simulink model "zoom_adc_single_pol_no_pfb"  (Simulink 7.8)
settings: Solver=FixedStepDiscrete  SolverMode=SingleTasking  StartTime=0.0  StopTime=4096  RelTol=1e-3
libraries: xbsIndex_r4 x331, xps_library x33, casper_library_misc x29, casper_library_delays x2, xbsReplacements_r4 x2, simulink x1, gavrt_library x1, casper_library_sources x1
(part 1 of 4)
settings Model: Creator=jayanth  LastModifiedBy=dprice  ModifiedComment=dprice -- Mon Apr 22 12:22:19 2013 -- Version 1.134
settings Model: ModifiedHistory=|
  | dprice -- Mon Apr 22 12:22:19 2013 -- Version 1.134
  | 
  | 
  | dprice -- Mon Apr 22 12:19:46 2013 -- Version 1.133
  | 
  | Removing PFB to see what happens
  | 
  | dprice -- Mon Apr 22 11:47:52 2013 -- Version 1.132
  | 
  | Trying pfb_fir_real
  | 
  | dprice -- Mon Apr 22 10:52:06 2013 -- Version 1.131
  | 
  | Reworked packetization part
  | 
  | dprice -- Mon Apr 22 08:09:55 2013 -- Version 1.130
  | 
  | Changed PFB window to flat top, 8 taps
  | 
  | dprice -- Sun Apr 21 17:53:15 2013 -- Version 1.129
  | 
  | Happy with the design. Need to be careful with bit overflows however, make sure fft_shift and cplx_quant_gain are set to reasonable levels using the snap blocks.
  | 
  | dprice -- Sun Apr 21 16:38:47 2013 -- Version 1.128
  | 
  | Please god work
  | 
  | dprice -- Sun Apr 21 16:02:02 2013 -- Version 1.127
  | 
  | Arrgghhhh
  | 
  | dprice -- Sun Apr 21 15:53:36 2013 -- Version 1.126
  | 
  | Trying new convert blocks
  | 
  | dprice -- Sun Apr 21 15:21:10 2013 -- Version 1.125
  | 
  | Added in digital noise source
  | 
  | dprice -- Sun Apr 21 14:43:26 2013 -- Version 1.124
  | 
  | Just work goddamit
  | 
  | dprice -- Sun Apr 21 13:10:26 2013 -- Version 1.123
  | 
  | Added unsigned snap block to quant
  | 
  | dprice -- Sun Apr 21 12:06:49 2013 -- Version 1.122
  | 
  | 
  | dprice -- Sun Apr 21 11:54:51 2013 -- Version 1.121
  | 
  | Changed over to quant block, added snap_quant for debugging
  | 
  | dprice -- Sun Apr 21 11:40:57 2013 -- Version 1.120
  | 
  | 
  | dprice -- Sun Apr 21 10:53:59 2013 -- Version 1.119
  | 
  | pkt_en going high now resets the packet counter in the header, and resets the 10 GbE core.
  | 
  | dprice -- Sat Apr 20 15:05:59 2013 -- Version 1.118
  | 
  | Fixed slice bug
  | 
  | dprice -- Sat Apr 20 14:12:55 2013 -- Version 1.117
  | 
  | Added a snap block after the quant
  | 
  | dprice -- Sat Apr 20 14:02:46 2013 -- Version 1.116
  | 
  | Trying to stop malformed packet being sent. Added in lots of pkt_en checks, so data is only valid when pkt_en is high
  | 
  | dprice -- Fri Apr 19 10:47:57 2013 -- Version 1.115
  | 
  | Switching to black boxed fft
settings DebuggingCC: FcnCallInpInsideContextMsg=Use local settings

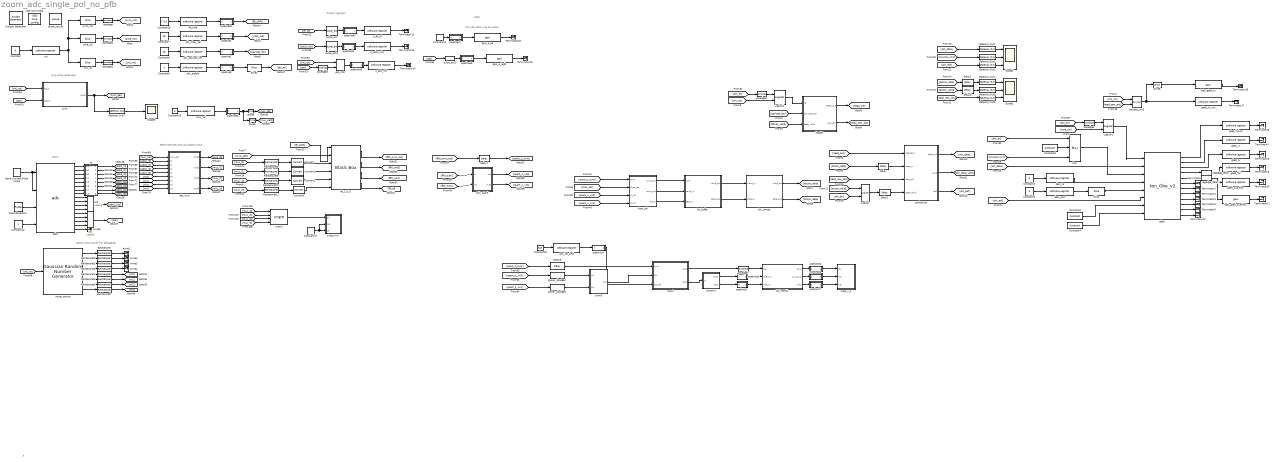
== system zoom_adc_single_pol_no_pfb ==
  note: Sync pulse reset logic
  note: ADC0
  note: Output registers
  note: this sets GPIO A as an output
  note: GPIO
  note: a
  note: Digital noise source (for debugging)
  note: Select between ADC and digital noise
  " System Generator" : xbsIndex_r4/ System Generator  Tag=genX  xilinxfamily=virtex5  part=xc5vsx95t  speed=-1  package=ff1136  synthesis_tool=XST  clock_wrapper=Clock Enables  directory=./zoom_adc_single_pol2/sysgen  proj_type=Project Navigator  Synth_file=XST Defaults  Impl_file=ISE Defaults  testbench=off  simulink_period=1  sysclk_period=5  dcm_input_clock_period=100  incr_netlist=off  trim_vbits=Everywhere in SubSystem  dbl_ovrd=According to Block Masks  core_generation=According to Block Masks  run_coregen=off  deprecated_control=off  eval_field=0  block_type=sysgen
  Arnie{1..4,9} (5 alike) : Terminator
  Band-Limited White Noise : simulink/Sources/Band-Limited White Noise  Cov=[0.1]  Ts=0.1  seed=[23341]
  Constant{,1..2,13} (4 alike) : Constant  Value=0
  Constant10 : Constant
  Constant14 : xbsIndex_r4/Constant  const=0  gui_display_data_type=Boolean  arith_type=Boolean  n_bits=16  bin_pt=14  preci_type=Single  exp_width=8  frac_width=24  explicit_period=on  period=1  equ=P=C  opselect=C  inp2=PCIN>>17  opr=+  inp1=P  carry=CIN  dbl_ovrd=off  block_type=constant
  Constant16 : Constant  Value=512
  Constant17 : xbsIndex_r4/Constant  const=1  gui_display_data_type=Boolean  arith_type=Boolean  n_bits=16  bin_pt=14  preci_type=Single  exp_width=8  frac_width=24  explicit_period=on  period=1  equ=P=C  opselect=C  inp2=PCIN>>17  opr=+  inp1=P  carry=CIN  dbl_ovrd=off  block_type=constant
  Constant18 : Constant  Value=2^5-1
  Constant3 : xbsIndex_r4/Constant  const=1  gui_display_data_type=Boolean  arith_type=Boolean  n_bits=16  bin_pt=14  preci_type=Single  exp_width=8  frac_width=24  explicit_period=on  period=1  equ=P=C  opselect=C  inp2=PCIN>>17  opr=+  inp1=P  carry=CIN  dbl_ovrd=off  block_type=constant
  Constant4 : xbsIndex_r4/Constant  const=0  gui_display_data_type=Boolean  arith_type=Boolean  n_bits=16  bin_pt=14  preci_type=Single  exp_width=8  frac_width=24  explicit_period=on  period=1  equ=P=C  opselect=C  inp2=PCIN>>17  opr=+  inp1=P  carry=CIN  dbl_ovrd=off  block_type=constant
  Constant5 : Constant
  Constant6 : xbsIndex_r4/Constant  const=0  gui_display_data_type=Boolean  arith_type=Boolean  n_bits=16  bin_pt=14  preci_type=Single  exp_width=8  frac_width=24  explicit_period=off  period=1  equ=P=C  opselect=C  inp2=PCIN>>17  opr=+  inp1=P  carry=CIN  dbl_ovrd=off  block_type=constant
  Constant7 : Constant  Value=15
  Constant8 : Constant  Value=16
  Convert{,1..3} (4 alike) : xbsIndex_r4/Convert  gui_display_data_type=Fixed-point  arith_type="Signed  (2's comp)"  n_bits=18  bin_pt=0  float_type=Single  exp_bits=8  fraction_bits=24  quantization=Truncate  overflow=Wrap  en=off  latency=0  dbl_ovrd=off  pipeline=off  block_type=convert
  Delay{,1..3} (4 alike) : xbsIndex_r4/Delay  rst=off  infoeditControl=Selection of Reset will increase slice count due to use of real FFs and instead of SRLs  en=off  latency=1  dbl_ovrd=off  reg_retiming=off  block_type=delay
  Delay6 : xbsIndex_r4/Delay  rst=off  infoeditControl=Selection of Reset will increase slice count due to use of real FFs and instead of SRLs  en=off  latency=4  dbl_ovrd=off  reg_retiming=off  block_type=delay
  Delay7 : xbsIndex_r4/Delay  rst=off  infoeditControl=Selection of Reset will increase slice count due to use of real FFs and instead of SRLs  en=off  latency=6  dbl_ovrd=off  reg_retiming=off  block_type=delay
  Gateway Out{1..7} (7 alike) : xbsIndex_r4/Gateway Out  inherit_from_input=off  hdl_port=on  timing_constraint=None  locs_specified=off  LOCs={}  UseAsDAC=off  DACChannel='1'  block_type=gatewayout
      settings: infoedit="Gateway out block.  Converts Xilinx fixed-point or floating-point type inputs into ouputs of type Simulink integer, single, double, or fixed point.<br><br>Hardware notes:  In hardware these blocks become top level output ports or are discarded, depending on how they are configured."
  Logical : xbsIndex_r4/Logical  logical_function=AND  inputs=2  en=off  latency=0  precision=Full  arith_type=Unsigned  n_bits=16  bin_pt=0  align_bp=on  dbl_ovrd=off  block_type=logical
  Logical1 : xbsIndex_r4/Logical  logical_function=OR  inputs=2  en=off  latency=0  precision=Full  arith_type=Unsigned  n_bits=16  bin_pt=0  align_bp=on  dbl_ovrd=off  block_type=logical
  Logical2 : xbsIndex_r4/Logical  logical_function=OR  inputs=2  en=off  latency=0  precision=Full  arith_type=Unsigned  n_bits=16  bin_pt=0  align_bp=on  dbl_ovrd=off  block_type=logical
  Mux : xbsIndex_r4/Mux  inputs=2  en=off  latency=0  precision=Full  arith_type=Unsigned  n_bits=16  bin_pt=14  quantization=Truncate  overflow=Wrap  dbl_ovrd=off  block_type=mux
  Pulse Generator : DiscretePulseGenerator  PulseType=Time based  Period=10  PulseWidth=5
  Reinterpret{,1..7} (8 alike) : xbsIndex_r4/Reinterpret  force_arith_type=on  arith_type="Signed  (2's comp)"  force_bin_pt=on  bin_pt=7  block_type=reinterpret
  Reinterpret{8..11} (4 alike) : xbsIndex_r4/Reinterpret  force_arith_type=on  arith_type="Signed  (2's comp)"  force_bin_pt=on  bin_pt=0  block_type=reinterpret
  Scope1 : Scope  NumInputPorts=3
      settings: TimeRange=290.1459854014598  YMin=9.44473e+21~0~-1  YMax=3.5e+38~1~1
  Scope2 : Scope  NumInputPorts=3
      settings: TimeRange=290.1459854014598  YMin=0~0~0  YMax=1.2e+18~1~1
  Scope3 : Scope
      settings: SaveName=ScopeData6
  Slice : xbsIndex_r4/Slice  nbits=16  boolean_output=off  mode=Lower Bit Location + Width  bit1=0  base1=MSB of Input  bit0=0  base0=LSB of Input  dbl_ovrd=off  block_type=slice
  Slice1 : xbsIndex_r4/Slice  nbits=1  boolean_output=on  mode=Lower Bit Location + Width  bit1=1  base1=MSB of Input  bit0=0  base0=LSB of Input  dbl_ovrd=off  block_type=slice
      settings: block_version=10.1.3
  Slice3 : xbsIndex_r4/Slice  nbits=1  boolean_output=on  mode=Lower Bit Location + Width  bit1=0  base1=MSB of Input  bit0=0  base0=LSB of Input  dbl_ovrd=off  block_type=slice
      settings: infoedit="Extracts a given range of bits from each input sample and presents it at the output.  The output type is ordinarily unsigned with binary point at zero, but can be Boolean when the slice is one bit wide.<P><P>Hardware notes: In hardware this block costs nothing."  block_version=10.1.3
  Slice5 : xbsIndex_r4/Slice  nbits=1  boolean_output=on  mode=Lower Bit Location + Width  bit1=0  base1=MSB of Input  bit0=1  base0=LSB of Input  dbl_ovrd=off  block_type=slice
      settings: block_version=10.1.3
  Slice6 : xbsIndex_r4/Slice  nbits=1  boolean_output=on  mode=Lower Bit Location + Width  bit1=0  base1=MSB of Input  bit0=15  base0=LSB of Input  dbl_ovrd=off  block_type=slice
      settings: block_version=10.1.3
  Terminator{,1..19} (20 alike) : Terminator
  XSG core config : xps_library/XSG core config  Tag=xps:xsg  hw_sys=ROACH:sx95t  ibob_linux=off  clk_src=adc0_clk  iBOB_clk_src=sys_clk  BEE2_ctrl_clk_src=sys_clk  BEE2_usr_clk_src=sys_clk  ROACH_clk_src=adc0_clk  ROACH2_clk_src=adc0_clk  CORR_clk_src=sys_clk  gpio_clk_io_group=iBOB:sma  gpio_clk_bit_index=0  clk_rate=200  sample_period=1  synthesis_tool=XST  mpc_type=powerpc440_ext
  acc_len_test : xps_library/software register  Tag=xps:sw_reg  io_dir=From Processor  arith_type=Unsigned  bitwidth=32  bin_pt=0  sample_period=1  gw_name=zoom_adc_single_pol2_acc_len_test_user_data_out
      link override coarse_r01_acc_len_user_data_out: arith_type=Unsigned  n_bits=32  bin_pt=0  period=1
      link override coarse_r02_acc_len_user_data_out: arith_type=Unsigned  n_bits=32  bin_pt=0  period=1
      link override coarse_r03_acc_len_user_data_out: arith_type=Unsigned  n_bits=32  bin_pt=0  period=1
      link override coarse_r04_acc_len_user_data_out: arith_type=Unsigned  n_bits=32  bin_pt=0  period=1
      link override coarse_r06_acc_len_user_data_out: arith_type=Unsigned  n_bits=32  bin_pt=0  period=1
      link override coarse_r07_acc_len_user_data_out: arith_type=Unsigned  n_bits=32  bin_pt=0  period=1
      link override coarse_r08_acc_len_user_data_out: arith_type=Unsigned  n_bits=32  bin_pt=0  period=1
      link override coarse_r09_acc_len_user_data_out: arith_type=Unsigned  n_bits=32  bin_pt=0  period=1
      link override coarse_r10_acc_len_user_data_out: arith_type=Unsigned  n_bits=32  bin_pt=0  period=1
      link override coarse_r12_acc_len_user_data_out: arith_type=Unsigned  n_bits=32  bin_pt=0  period=1
      link override convert: arith_type=Unsigned  n_bits=32  bin_pt=0
      link override qdr_single_r01_acc_len_coarse_user_data_out: arith_type=Unsigned  n_bits=32  bin_pt=0  period=1
      link override qdr_single_r02_acc_len_coarse_user_data_out: arith_type=Unsigned  n_bits=32  bin_pt=0  period=1
      link override qdr_single_r04_acc_len_coarse_user_data_out: arith_type=Unsigned  n_bits=32  bin_pt=0  period=1
      link override r_spec_2048_r102_acc_len_user_data_out: arith_type=Unsigned  n_bits=32  bin_pt=0  period=1
      link override r_spec_2048_r102_nosim_acc_len_user_data_out: arith_type=Unsigned  n_bits=32  bin_pt=0  period=1
      link override r_spec_2048_r103_acc_len_user_data_out: arith_type=Unsigned  n_bits=32  bin_pt=0  period=1
      link override r_spec_2048_r104_acc_len_user_data_out: arith_type=Unsigned  n_bits=32  bin_pt=0  period=1
      link override r_spec_2048_r104_test0_acc_len_user_data_out: arith_type=Unsigned  n_bits=32  bin_pt=0  period=1
      link override r_spec_2048_r104_test_acc_len_user_data_out: arith_type=Unsigned  n_bits=32  bin_pt=0  period=1
      link override r_spec_2048_r105_acc_len_user_data_out: arith_type=Unsigned  n_bits=32  bin_pt=0  period=1
      link override r_spec_test0_acc_len_user_data_out: arith_type=Unsigned  n_bits=32  bin_pt=0  period=1
      link override r_spec_test4_acc_len_user_data_out: arith_type=Unsigned  n_bits=32  bin_pt=0  period=1
      link override roach_spec_acc_len_user_data_out: arith_type=Unsigned  n_bits=32  bin_pt=0  period=1
      link override roach_spec_no10gbe_acc_len_user_data_out: arith_type=Unsigned  n_bits=32  bin_pt=0  period=1
      link override roach_spec_rst_sync_cnt_user_data_out: arith_type=Unsigned  n_bits=32  bin_pt=0  period=1
      link override toast_256ch_16bit_r02_acc_len_user_data_out: arith_type=Unsigned  n_bits=32  bin_pt=0  period=1
      link override toast_256ch_16bit_r04_acc_len_user_data_out: arith_type=Unsigned  n_bits=32  bin_pt=0  period=1
      link override toast_256ch_16bit_r05_acc_len_user_data_out: arith_type=Unsigned  n_bits=32  bin_pt=0  period=1
  adc0 : xps_library/adc  Tag=xps:adc  adc_brd=adc0  adc_clk_rate=800  adc_interleave=off  sample_period=1
  adc_mux : Subsystem
  bit_buffer : Subsystem
  bit_concat : Subsystem
  buf_reord1 : Subsystem  mask{buf_len_bits=3}
      mask prompts: buf_len_bits "Buffer length (2^N)"
      mask help: Concatenate signals together for snap block
  chan_sel : Subsystem
  cnt_rst : xbsIndex_r4/Slice  nbits=1  boolean_output=on  mode=Lower Bit Location + Width  bit1=0  base1=MSB of Input  bit0=0  base0=LSB of Input  dbl_ovrd=off  block_type=slice
  core_rst : xbsIndex_r4/Slice  nbits=1  boolean_output=on  mode=Lower Bit Location + Width  bit1=0  base1=MSB of Input  bit0=1  base0=LSB of Input  dbl_ovrd=off  block_type=slice
  cplx_quant : Subsystem  AncestorBlock=hipsr_library/quant
  cram1 : gavrt_library/cram  num_slice=4
  cram5 : Subsystem  AncestorBlock=gavrt_library/cram  mask(cram){num_slice=2}
      mask prompts: num_slice "Number of slices"
      mask help: Concatenates inputs together after forcing binary point to zero.
      mask init:
      | cram_init(gcb, ...
      |     'num_slice', num_slice);
  dest_ip : xps_library/software register  Tag=xps:sw_reg  io_dir=From Processor  arith_type=Unsigned  bitwidth=32  bin_pt=0  sample_period=1  gw_name=zoom_adc_single_pol2_dest_ip_user_data_out
      link override hipsr_tgbe3_dest_ip_user_data_out: arith_type=Unsigned  n_bits=32  bin_pt=0  period=1
  dest_port : xps_library/software register  Tag=xps:sw_reg  io_dir=From Processor  arith_type=Unsigned  bitwidth=32  bin_pt=0  sample_period=1  gw_name=zoom_adc_single_pol2_dest_port_user_data_out
      link override hipsr_tgbe3_dest_port_user_data_out: arith_type=Unsigned  n_bits=32  bin_pt=0  period=1
  fft_11x_0 : xbsIndex_r4/Black Box  init_code=fft_32pt_11x_core_config  sim_method=Inactive  verbose=off  block_type=blackbox2
  fft_shift : xps_library/software register  Tag=xps:sw_reg  io_dir=From Processor  arith_type=Unsigned  bitwidth=32  bin_pt=0  sample_period=1  gw_name=zoom_adc_single_pol2_fft_shift_user_data_out
      link override coarse_r01_acc_len_user_data_out: arith_type=Unsigned  n_bits=32  bin_pt=0  period=1
      link override coarse_r02_acc_len_user_data_out: arith_type=Unsigned  n_bits=32  bin_pt=0  period=1
      link override coarse_r03_acc_len_user_data_out: arith_type=Unsigned  n_bits=32  bin_pt=0  period=1
      link override coarse_r04_acc_len_user_data_out: arith_type=Unsigned  n_bits=32  bin_pt=0  period=1
      link override coarse_r06_acc_len_user_data_out: arith_type=Unsigned  n_bits=32  bin_pt=0  period=1
      link override coarse_r07_acc_len_user_data_out: arith_type=Unsigned  n_bits=32  bin_pt=0  period=1
      link override coarse_r08_acc_len_user_data_out: arith_type=Unsigned  n_bits=32  bin_pt=0  period=1
      link override coarse_r09_acc_len_user_data_out: arith_type=Unsigned  n_bits=32  bin_pt=0  period=1
      link override coarse_r10_acc_len_user_data_out: arith_type=Unsigned  n_bits=32  bin_pt=0  period=1
      link override coarse_r12_acc_len_user_data_out: arith_type=Unsigned  n_bits=32  bin_pt=0  period=1
      link override convert: arith_type=Unsigned  n_bits=32  bin_pt=0
      link override qdr_single_r01_acc_len_coarse_user_data_out: arith_type=Unsigned  n_bits=32  bin_pt=0  period=1
      link override qdr_single_r02_acc_len_coarse_user_data_out: arith_type=Unsigned  n_bits=32  bin_pt=0  period=1
      link override qdr_single_r04_acc_len_coarse_user_data_out: arith_type=Unsigned  n_bits=32  bin_pt=0  period=1
      link override r_spec_2048_r102_acc_len_user_data_out: arith_type=Unsigned  n_bits=32  bin_pt=0  period=1
      link override r_spec_2048_r102_nosim_acc_len_user_data_out: arith_type=Unsigned  n_bits=32  bin_pt=0  period=1
      link override r_spec_2048_r103_acc_len_user_data_out: arith_type=Unsigned  n_bits=32  bin_pt=0  period=1
      link override r_spec_2048_r104_acc_len_user_data_out: arith_type=Unsigned  n_bits=32  bin_pt=0  period=1
      link override r_spec_2048_r104_test0_acc_len_user_data_out: arith_type=Unsigned  n_bits=32  bin_pt=0  period=1
      link override r_spec_2048_r104_test_acc_len_user_data_out: arith_type=Unsigned  n_bits=32  bin_pt=0  period=1
      link override r_spec_2048_r105_acc_len_user_data_out: arith_type=Unsigned  n_bits=32  bin_pt=0  period=1
      link override r_spec_test0_acc_len_user_data_out: arith_type=Unsigned  n_bits=32  bin_pt=0  period=1
      link override r_spec_test4_acc_len_user_data_out: arith_type=Unsigned  n_bits=32  bin_pt=0  period=1
      link override roach_spec_acc_len_user_data_out: arith_type=Unsigned  n_bits=32  bin_pt=0  period=1
      link override roach_spec_no10gbe_acc_len_user_data_out: arith_type=Unsigned  n_bits=32  bin_pt=0  period=1
      link override roach_spec_rst_sync_cnt_user_data_out: arith_type=Unsigned  n_bits=32  bin_pt=0  period=1
      link override toast_256ch_16bit_r02_acc_len_user_data_out: arith_type=Unsigned  n_bits=32  bin_pt=0  period=1
      link override toast_256ch_16bit_r04_acc_len_user_data_out: arith_type=Unsigned  n_bits=32  bin_pt=0  period=1
      link override toast_256ch_16bit_r05_acc_len_user_data_out: arith_type=Unsigned  n_bits=32  bin_pt=0  period=1
  gbe0 : xps_library/ten_Gbe_v2  Tag=xps:tengbe_v2  port=0  rx_dist_ram=off  large_frames=on  show_param=off  pre_emph=3  swing=800  fab_en=on  fab_mac=hex2dec('123456780000')  fab_ip=192*(2^24) + 168*(2^16) + 5*(2^8) + 20*(2^0)  fab_udp=10000  fab_gate=1  cpu_rx_en=on  cpu_tx_en=on
  gbe0_full : xps_library/software register  Tag=xps:sw_reg  io_dir=To Processor  arith_type=Unsigned  bitwidth=32  bin_pt=0  sample_period=1  gw_name=zoom_adc_single_pol2_gbe0_full_user_data_in
      link override convert: arith_type=Unsigned  n_bits=32  bin_pt=0
  gbe0_linkup : xps_library/software register  Tag=xps:sw_reg  io_dir=To Processor  arith_type=Unsigned  bitwidth=32  bin_pt=0  sample_period=1  gw_name=zoom_adc_single_pol2_gbe0_linkup_user_data_in
      link override convert: arith_type=Unsigned  n_bits=32  bin_pt=0
  gbe0_overflow : xps_library/software register  Tag=xps:sw_reg  io_dir=To Processor  arith_type=Unsigned  bitwidth=32  bin_pt=0  sample_period=1  gw_name=zoom_adc_single_pol2_gbe0_overflow_user_data_in
      link override convert: arith_type=Unsigned  n_bits=32  bin_pt=0
  gbe0_rx : xps_library/software register  Tag=xps:sw_reg  io_dir=To Processor  arith_type=Unsigned  bitwidth=32  bin_pt=0  sample_period=1  gw_name=zoom_adc_single_pol2_gbe0_rx_user_data_in
      link override convert: arith_type=Unsigned  n_bits=32  bin_pt=0
  gbe0_tx : xps_library/software register  Tag=xps:sw_reg  io_dir=To Processor  arith_type=Unsigned  bitwidth=32  bin_pt=0  sample_period=1  gw_name=zoom_adc_single_pol2_gbe0_tx_user_data_in
      link override convert: arith_type=Unsigned  n_bits=32  bin_pt=0
  gbe0_tx_cnt : xps_library/software register  Tag=xps:sw_reg  io_dir=To Processor  arith_type=Unsigned  bitwidth=32  bin_pt=0  sample_period=1  gw_name=zoom_adc_single_pol2_gbe0_tx_cnt_user_data_in
      link override convert: arith_type=Unsigned  n_bits=32  bin_pt=0
  gpio_a_led0 : xps_library/gpio  Tag=xps:gpio  io_group=ROACH:gpioa  io_dir=out  arith_type=Boolean  bitwidth=1  bin_pt=0  bit_index=4  sample_period=1  use_single_ended=off  use_ddr=off  reg_iob=on  reg_clk_phase=0  termination=None
  gpio_a_oe : xps_library/gpio  Tag=xps:gpio  io_group=ROACH:gpioa_oe_n  io_dir=out  arith_type=Boolean  bitwidth=1  bin_pt=0  bit_index=0  sample_period=1  use_single_ended=off  use_ddr=off  reg_iob=on  reg_clk_phase=0  termination=None
  header : Subsystem
  led0_gbe0_overflow : xps_library/gpio  Tag=xps:gpio  io_group=ROACH:led  io_dir=out  arith_type=Boolean  bitwidth=1  bin_pt=0  bit_index=1  sample_period=1  use_single_ended=off  use_ddr=off  reg_iob=on  reg_clk_phase=0  termination=None
      link override convert: arith_type=Boolean  n_bits=1  bin_pt=0
  led0_gbe0_tx : xps_library/gpio  Tag=xps:gpio  io_group=ROACH:led  io_dir=out  arith_type=Boolean  bitwidth=1  bin_pt=0  bit_index=0  sample_period=1  use_single_ended=off  use_ddr=off  reg_iob=off  reg_clk_phase=0  termination=None
      link override convert: arith_type=Boolean  n_bits=1  bin_pt=0
  mux_sel : xps_library/software register  Tag=xps:sw_reg  io_dir=From Processor  arith_type=Unsigned  bitwidth=32  bin_pt=0  sample_period=1  gw_name=zoom_adc_single_pol2_mux_sel_user_data_out
      link override coarse_r01_mux_sel_user_data_out: arith_type=Unsigned  n_bits=32  bin_pt=0  period=1
      link override coarse_r02_mux_sel_user_data_out: arith_type=Unsigned  n_bits=32  bin_pt=0  period=1
      link override coarse_r03_mux_sel_user_data_out: arith_type=Unsigned  n_bits=32  bin_pt=0  period=1
      link override coarse_r04_mux_sel_user_data_out: arith_type=Unsigned  n_bits=32  bin_pt=0  period=1
      link override coarse_r06_mux_sel_user_data_out: arith_type=Unsigned  n_bits=32  bin_pt=0  period=1
      link override coarse_r07_mux_sel_user_data_out: arith_type=Unsigned  n_bits=32  bin_pt=0  period=1
      link override coarse_r08_mux_sel_user_data_out: arith_type=Unsigned  n_bits=32  bin_pt=0  period=1
      link override coarse_r09_mux_sel_user_data_out: arith_type=Unsigned  n_bits=32  bin_pt=0  period=1
      link override coarse_r10_mux_sel_user_data_out: arith_type=Unsigned  n_bits=32  bin_pt=0  period=1
      link override coarse_r12_mux_sel_user_data_out: arith_type=Unsigned  n_bits=32  bin_pt=0  period=1
      link override convert: arith_type=Unsigned  n_bits=32  bin_pt=0
      link override qdr_single_r01_mux_sel_user_data_out: arith_type=Unsigned  n_bits=32  bin_pt=0  period=1
      link override qdr_single_r02_mux_sel_user_data_out: arith_type=Unsigned  n_bits=32  bin_pt=0  period=1
      link override qdr_single_r04_mux_sel_user_data_out: arith_type=Unsigned  n_bits=32  bin_pt=0  period=1
      link override r_spec_2048_r102_cnt_rst_user_data_out: arith_type=Unsigned  n_bits=32  bin_pt=0  period=1
      link override r_spec_2048_r102_nosim_cnt_rst_user_data_out: arith_type=Unsigned  n_bits=32  bin_pt=0  period=1
      link override r_spec_2048_r103_cnt_rst_user_data_out: arith_type=Unsigned  n_bits=32  bin_pt=0  period=1
      link override r_spec_2048_r104_cnt_rst_user_data_out: arith_type=Unsigned  n_bits=32  bin_pt=0  period=1
      link override r_spec_2048_r104_test0_cnt_rst_user_data_out: arith_type=Unsigned  n_bits=32  bin_pt=0  period=1
      link override r_spec_2048_r104_test_cnt_rst_user_data_out: arith_type=Unsigned  n_bits=32  bin_pt=0  period=1
      link override r_spec_2048_r105_cnt_rst_user_data_out: arith_type=Unsigned  n_bits=32  bin_pt=0  period=1
      link override r_spec_test0_cnt_rst_user_data_out: arith_type=Unsigned  n_bits=32  bin_pt=0  period=1
      link override r_spec_test4_cnt_rst_user_data_out: arith_type=Unsigned  n_bits=32  bin_pt=0  period=1
      link override roach_spec_no10gbe_sync_cnt_rst_user_data_out: arith_type=Unsigned  n_bits=32  bin_pt=0  period=1
      link override roach_spec_rst_cnt_user_data_out: arith_type=Unsigned  n_bits=32  bin_pt=0  period=1
      link override roach_spec_rst_sync_cnt_user_data_out: arith_type=Unsigned  n_bits=32  bin_pt=0  period=1
      link override toast_256ch_16bit_r02_cnt_rst_user_data_out: arith_type=Unsigned  n_bits=32  bin_pt=0  period=1
      link override toast_256ch_16bit_r04_cnt_rst_user_data_out: arith_type=Unsigned  n_bits=32  bin_pt=0  period=1
      link override toast_256ch_16bit_r05_cnt_rst_user_data_out: arith_type=Unsigned  n_bits=32  bin_pt=0  period=1
  negedge_delay : casper_library_misc/negedge_delay  pulse_len=2^20
  negedge_delay1 : casper_library_misc/negedge_delay  pulse_len=2^20
  noise_source : casper_library_sources/Gaussian Random Number Generator
  o_adc0_clip : xps_library/software register  Tag=xps:sw_reg  io_dir=To Processor  arith_type=Unsigned  bitwidth=32  bin_pt=0  sample_period=1  gw_name=zoom_adc_single_pol2_o_adc0_clip_user_data_in
      link override convert: arith_type=Unsigned  n_bits=32  bin_pt=0
  o_fft_of : xps_library/software register  Tag=xps:sw_reg  io_dir=To Processor  arith_type=Unsigned  bitwidth=32  bin_pt=0  sample_period=1  gw_name=zoom_adc_single_pol2_o_fft_of_user_data_in
      link override convert: arith_type=Unsigned  n_bits=32  bin_pt=0
  o_pps_cnt : xps_library/software register  Tag=xps:sw_reg  io_dir=To Processor  arith_type=Unsigned  bitwidth=32  bin_pt=0  sample_period=1  gw_name=zoom_adc_single_pol2_o_pps_cnt_user_data_in
      link override convert: arith_type=Unsigned  n_bits=32  bin_pt=0
  or0 : xbsIndex_r4/Logical  logical_function=OR  inputs=4  en=off  latency=2  precision=Full  arith_type=Unsigned  n_bits=16  bin_pt=0  align_bp=off  dbl_ovrd=off  block_type=logical
  or1 : xbsIndex_r4/Logical  logical_function=OR  inputs=4  en=off  latency=2  precision=Full  arith_type=Unsigned  n_bits=16  bin_pt=0  align_bp=off  dbl_ovrd=off  block_type=logical
  packetizer : Subsystem
  payload_cnt5 : xbsIndex_r4/Counter  cnt_type=Free Running  cnt_to=0  operation=Up  start_count=0  cnt_by_val=1  arith_type=Unsigned  n_bits=32  bin_pt=0  load_pin=off  rst=on  en=on  explicit_period=on  period=1  dbl_ovrd=off  use_behavioral_HDL=off  implementation=Fabric  use_rpm=on  block_type=counter
      settings: xl_area=[0, 0, 0, 0, 0, 0, 0]
  pcore_nar_fft : xps_library/pcore  Tag=xps:pcore  pcore_path=./bb/fft_32pt_11x_core/fft_32pt_11x_core.ngc
  pipeline1 : Subsystem  AncestorBlock=casper_library_delays/pipeline  mask(pipeline){latency=1}
      mask help:
      | An explicitly laid-out delay line to avoid mapping to SRL16s
      | for use in pipelining to help achieve timing closure.
      mask init:
      | pipeline_init(gcb,'latency',latency);
      settings: AttributesFormatString=Latency = %<latency>
      mask Display:
      | port_label('input', 1, 'd');
      | port_label('output', 1, 'q');
      | disp(['Z^-', num2str(latency)]);
  pipeline14 : Subsystem  AncestorBlock=casper_library_delays/pipeline  mask(pipeline){latency=1}
      mask help:
      | An explicitly laid-out delay line to avoid mapping to SRL16s
      | for use in pipelining to help achieve timing closure.
      mask init:
      | pipeline_init(gcb,'latency',latency);
      settings: AttributesFormatString=Latency = %<latency>
      mask Display:
      | port_label('input', 1, 'd');
      | port_label('output', 1, 'q');
      | disp(['Z^-', num2str(latency)]);
  pipeline15 : Subsystem  AncestorBlock=casper_library_delays/pipeline  mask(pipeline){latency=1}
      mask help:
      | An explicitly laid-out delay line to avoid mapping to SRL16s
      | for use in pipelining to help achieve timing closure.
      mask init:
      | pipeline_init(gcb,'latency',latency);
      settings: AttributesFormatString=Latency = %<latency>
      mask Display:
      | port_label('input', 1, 'd');
      | port_label('output', 1, 'q');
      | disp(['Z^-', num2str(latency)]);
  pipeline17 : Subsystem  AncestorBlock=casper_library_delays/pipeline  mask(pipeline){latency=1}
      mask help:
      | An explicitly laid-out delay line to avoid mapping to SRL16s
      | for use in pipelining to help achieve timing closure.
      mask init:
      | pipeline_init(gcb,'latency',latency);
      settings: AttributesFormatString=Latency = %<latency>
      mask Display:
      | port_label('input', 1, 'd');
      | port_label('output', 1, 'q');
      | disp(['Z^-', num2str(latency)]);
  pipeline18 : Subsystem  AncestorBlock=casper_library_delays/pipeline  mask(pipeline){latency=1}
      mask help:
      | An explicitly laid-out delay line to avoid mapping to SRL16s
      | for use in pipelining to help achieve timing closure.
      mask init:
      | pipeline_init(gcb,'latency',latency);
      settings: AttributesFormatString=Latency = %<latency>
      mask Display:
      | port_label('input', 1, 'd');
      | port_label('output', 1, 'q');
      | disp(['Z^-', num2str(latency)]);
  pipeline2 : Subsystem  AncestorBlock=casper_library_delays/pipeline  mask(pipeline){latency=1}
      mask help:
      | An explicitly laid-out delay line to avoid mapping to SRL16s
      | for use in pipelining to help achieve timing closure.
      mask init:
      | pipeline_init(gcb,'latency',latency);
      settings: AttributesFormatString=Latency = %<latency>
      mask Display:
      | port_label('input', 1, 'd');
      | port_label('output', 1, 'q');
      | disp(['Z^-', num2str(latency)]);
  pipeline21 : Subsystem  AncestorBlock=casper_library_delays/pipeline  mask(pipeline){latency=1}
      mask help:
      | An explicitly laid-out delay line to avoid mapping to SRL16s
      | for use in pipelining to help achieve timing closure.
      mask init:
      | pipeline_init(gcb,'latency',latency);
      settings: AttributesFormatString=Latency = %<latency>
      mask Display:
      | port_label('input', 1, 'd');
      | port_label('output', 1, 'q');
      | disp(['Z^-', num2str(latency)]);
  pipeline22 : Subsystem  AncestorBlock=casper_library_delays/pipeline  mask(pipeline){latency=1}
      mask help:
      | An explicitly laid-out delay line to avoid mapping to SRL16s
      | for use in pipelining to help achieve timing closure.
      mask init:
      | pipeline_init(gcb,'latency',latency);
      settings: AttributesFormatString=Latency = %<latency>
      mask Display:
      | port_label('input', 1, 'd');
      | port_label('output', 1, 'q');
      | disp(['Z^-', num2str(latency)]);
  pipeline28 : Subsystem  AncestorBlock=casper_library_delays/pipeline  mask(pipeline){latency=1}
      mask help:
      | An explicitly laid-out delay line to avoid mapping to SRL16s
      | for use in pipelining to help achieve timing closure.
      mask init:
      | pipeline_init(gcb,'latency',latency);
      settings: AttributesFormatString=Latency = %<latency>
      mask Display:
      | port_label('input', 1, 'd');
      | port_label('output', 1, 'q');
      | disp(['Z^-', num2str(latency)]);
  pipeline3 : Subsystem  AncestorBlock=casper_library_delays/pipeline  mask(pipeline){latency=1}
      mask help:
      | An explicitly laid-out delay line to avoid mapping to SRL16s
      | for use in pipelining to help achieve timing closure.
      mask init:
      | pipeline_init(gcb,'latency',latency);
      settings: AttributesFormatString=Latency = %<latency>
      mask Display:
      | port_label('input', 1, 'd');
      | port_label('output', 1, 'q');
      | disp(['Z^-', num2str(latency)]);
  pipeline43 : Subsystem  AncestorBlock=casper_library_delays/pipeline  mask(pipeline){latency=1}
      mask help:
      | An explicitly laid-out delay line to avoid mapping to SRL16s
      | for use in pipelining to help achieve timing closure.
      mask init:
      | pipeline_init(gcb,'latency',latency);
      settings: AttributesFormatString=Latency = %<latency>
      mask Display:
      | port_label('input', 1, 'd');
      | port_label('output', 1, 'q');
      | disp(['Z^-', num2str(latency)]);
  pipeline44 : Subsystem  AncestorBlock=casper_library_delays/pipeline  mask(pipeline){latency=1}
      mask help:
      | An explicitly laid-out delay line to avoid mapping to SRL16s
      | for use in pipelining to help achieve timing closure.
      mask init:
      | pipeline_init(gcb,'latency',latency);
      settings: AttributesFormatString=Latency = %<latency>
      mask Display:
      | port_label('input', 1, 'd');
      | port_label('output', 1, 'q');
      | disp(['Z^-', num2str(latency)]);
  pipeline46 : Subsystem  AncestorBlock=casper_library_delays/pipeline  mask(pipeline){latency=1}
      mask help:
      | An explicitly laid-out delay line to avoid mapping to SRL16s
      | for use in pipelining to help achieve timing closure.
      mask init:
      | pipeline_init(gcb,'latency',latency);
      settings: AttributesFormatString=Latency = %<latency>
      mask Display:
      | port_label('input', 1, 'd');
      | port_label('output', 1, 'q');
      | disp(['Z^-', num2str(latency)]);
  pipeline47 : Subsystem  AncestorBlock=casper_library_delays/pipeline  mask(pipeline){latency=1}
      mask help:
      | An explicitly laid-out delay line to avoid mapping to SRL16s
      | for use in pipelining to help achieve timing closure.
      mask init:
      | pipeline_init(gcb,'latency',latency);
      settings: AttributesFormatString=Latency = %<latency>
      mask Display:
      | port_label('input', 1, 'd');
      | port_label('output', 1, 'q');
      | disp(['Z^-', num2str(latency)]);
  pipeline48 : Subsystem  AncestorBlock=casper_library_delays/pipeline  mask(pipeline){latency=1}
      mask help:
      | An explicitly laid-out delay line to avoid mapping to SRL16s
      | for use in pipelining to help achieve timing closure.
      mask init:
      | pipeline_init(gcb,'latency',latency);
      settings: AttributesFormatString=Latency = %<latency>
      mask Display:
      | port_label('input', 1, 'd');
      | port_label('output', 1, 'q');
      | disp(['Z^-', num2str(latency)]);
  pipeline50 : Subsystem  AncestorBlock=casper_library_delays/pipeline  mask(pipeline){latency=1}
      mask help:
      | An explicitly laid-out delay line to avoid mapping to SRL16s
      | for use in pipelining to help achieve timing closure.
      mask init:
      | pipeline_init(gcb,'latency',latency);
      settings: AttributesFormatString=Latency = %<latency>
      mask Display:
      | port_label('input', 1, 'd');
      | port_label('output', 1, 'q');
      | disp(['Z^-', num2str(latency)]);
  pkt_chan_sel : xps_library/software register  Tag=xps:sw_reg  io_dir=From Processor  arith_type=Unsigned  bitwidth=32  bin_pt=0  sample_period=1  gw_name=zoom_adc_single_pol2_pkt_chan_sel_user_data_out
      link override hipsr_tgbe3_pkt_chan_sel_user_data_out: arith_type=Unsigned  n_bits=32  bin_pt=0  period=1
  pkt_enable : xps_library/software register  Tag=xps:sw_reg  io_dir=From Processor  arith_type=Unsigned  bitwidth=32  bin_pt=0  sample_period=1  gw_name=zoom_adc_single_pol2_pkt_enable_user_data_out
      link override hipsr_tgbe3_pkt_enable_user_data_out: arith_type=Unsigned  n_bits=32  bin_pt=0  period=1
  pkt_payload_len : xps_library/software register  Tag=xps:sw_reg  io_dir=From Processor  arith_type=Unsigned  bitwidth=32  bin_pt=0  sample_period=1  gw_name=zoom_adc_single_pol2_pkt_payload_len_user_data_out
      link override hipsr_tgbe3_pkt_payload_len_user_data_out: arith_type=Unsigned  n_bits=32  bin_pt=0  period=1
  pl : Subsystem
  posedge{,1..5,7} (7 alike) : casper_library_misc/posedge  Tag=Rising Edge Detector
  power_dsp48e2 : casper_library_misc/power_dsp48e  bitwidth=8
      link override slice_imag: nbits=bitwidth  boolean_output=off  mode=Lower Bit Location + Width  bit1=0  base1=MSB of Input  bit0=0  base0=LSB of Input  dbl_ovrd=off
      link override slice_real: nbits=bitwidth  boolean_output=off  mode=Upper Bit Location + Width  bit1=0  base1=MSB of Input  bit0=0  base0=LSB of Input  dbl_ovrd=off
      link override slice_imag: infoedit="Extracts a given range of bits from each input sample and presents it at the output.  The output type is ordinarily unsigned with binary point at zero, but can be Boolean when the slice is one bit wide.<br><br>Hardware notes: In hardware this block costs nothing."
      link override slice_real: infoedit="Extracts a given range of bits from each input sample and presents it at the output.  The output type is ordinarily unsigned with binary point at zero, but can be Boolean when the slice is one bit wide.<br><br>Hardware notes: In hardware this block costs nothing."
  power_dsp48e3 : casper_library_misc/power_dsp48e  bitwidth=8
      link override slice_imag: nbits=bitwidth  boolean_output=off  mode=Lower Bit Location + Width  bit1=0  base1=MSB of Input  bit0=0  base0=LSB of Input  dbl_ovrd=off
      link override slice_real: nbits=bitwidth  boolean_output=off  mode=Upper Bit Location + Width  bit1=0  base1=MSB of Input  bit0=0  base0=LSB of Input  dbl_ovrd=off
      link override slice_imag: infoedit="Extracts a given range of bits from each input sample and presents it at the output.  The output type is ordinarily unsigned with binary point at zero, but can be Boolean when the slice is one bit wide.<br><br>Hardware notes: In hardware this block costs nothing."
      link override slice_real: infoedit="Extracts a given range of bits from each input sample and presents it at the output.  The output type is ordinarily unsigned with binary point at zero, but can be Boolean when the slice is one bit wide.<br><br>Hardware notes: In hardware this block costs nothing."
  pps_cntr : xbsIndex_r4/Counter  cnt_type=Free Running  cnt_to=Inf  operation=Up  start_count=0  cnt_by_val=1  arith_type=Unsigned  n_bits=16  bin_pt=0  load_pin=off  rst=on  en=on  explicit_period=on  period=1  dbl_ovrd=off  use_behavioral_HDL=off  implementation=Fabric  use_rpm=off  block_type=counter
  pulse_ext : casper_library_misc/pulse_ext  pulse_len=100000
  pulse_ext2 : casper_library_misc/pulse_ext  pulse_len=2^24
  pulse_ext3 : casper_library_misc/pulse_ext  pulse_len=100000
  rst : xps_library/software register  Tag=xps:sw_reg  io_dir=From Processor  arith_type=Unsigned  bitwidth=32  bin_pt=0  sample_period=1  gw_name=zoom_adc_single_pol2_rst_user_data_out
      link override hipsr_tgbe3_rst_user_data_out: arith_type=Unsigned  n_bits=32  bin_pt=0  period=1
  snap_mux : Subsystem  AncestorBlock=casper_library_scopes/snap  mask(snap){nsamples=10}
      mask prompts: nsamples "No. of samples(2^?)"
      mask help:
      | The <i>snap</i> block provides a packaged solution to capturing data from
      | the FPGA fabric and making it accessible from the CPU.  The block comes in
      | 2 flavors: <i>snap</i> (which captures to a 32 bit wide Shared BRAM), and
      | <i>snap64</i> (which captures to 2x32 bit wide Shared BRAMs to effect a
      | 64 bit capture).
      mask Help: eval('xlWeb(''http://casper.berkeley.edu/wiki/Snap'')')
  snap_x_s : Subsystem  AncestorBlock=casper_library_scopes/snap  mask(snap){nsamples=10}
      mask prompts: nsamples "No. of samples(2^?)"
      mask help:
      | The <i>snap</i> block provides a packaged solution to capturing data from
      | the FPGA fabric and making it accessible from the CPU.  The block comes in
      | 2 flavors: <i>snap</i> (which captures to a 32 bit wide Shared BRAM), and
      | <i>snap64</i> (which captures to 2x32 bit wide Shared BRAMs to effect a
      | 64 bit capture).
      mask Help: eval('xlWeb(''http://casper.berkeley.edu/wiki/Snap'')')
  sync : Subsystem  AncestorBlock=hipsr_library/sync
  sync_rst : xbsIndex_r4/Slice  nbits=1  boolean_output=on  mode=Lower Bit Location + Width  bit1=0  base1=MSB of Input  bit0=2  base0=LSB of Input  dbl_ovrd=off  block_type=slice
  uncram1 : Subsystem  AncestorBlock=gavrt_library/uncram  mask(uncram){num_slice=2, slice_width=32, bin_pt=0, arith_type=0}
      mask prompts: num_slice "Number of slices"; slice_width "Slice Width"; bin_pt "Output Binary Point"; arith_type "Output Arithmetic Type (0=Unsigned, 1=Signed)"
      mask help: Takes a concatenated input and slices it up into even pieces and reinterprets them as signed fixed point numbers with a given binary point.
      mask init:
      | uncram_init(gcb, ...
      |     'num_slice', num_slice, ...
      |     'slice_width', slice_width, ...
      |     'bin_pt', bin_pt, ...
      |     'arith_type', arith_type);
      settings: AttributesFormatString=UFix_32_0
  vacc1 : Subsystem  AncestorBlock=gavrt_library/vacc  mask(vacc){veclen=3, ninputs=2, max_accum=27, arith_type=0, in_bit_width=16, in_bin_pt=0, out_bit_width=32, out_bin_pt=0, register_din=0, add_latency=2, bram_latency=2, mux_latency=1}
      mask prompts: veclen "Vector Length (2^?)"; ninputs "Number of Simultaneous Vectors"; max_accum "Maximum # Accumulations (2^?)"; arith_type "Arithmetic Type (0=Unsigned, 1=Signed)"; in_bit_width "Input Bit Width"; in_bin_pt "Input Binary Point"; out_bit_width "Output Bit Width"; out_bin_pt "Output Binary Point"; register_din "Register Input Data  (1 = Enable Register Retiming on input delay)"; add_latency "Add Latency"; bram_latency "BRAM Latency"; mux_latency "Mux Latency"
      mask help:
      | Sync is expected the clock before the first data to be accumulated.
      | Use the Cram block to feed din for multiple simultaneous inputs.
      | acc_len is the number of vectors to sum.
      | valid is high while a valid accumulation is being clocked out on dout.
      | If only one sync pulse is provided, valid accumulations will repeatedly appear on dout as they become available.
      | Undesired behavior may occur if acc_len is changed without a new sync pulse.
      | Note: The output is an unsigned concatenation of the output streams. Thus, even with only one stream, the output must be recast before using.
      | Register Input Data controls the "Enable Register Retiming" parameter of the delays on 'din'.
      mask init:
      | vacc_init(gcb, ...
      |     'veclen', veclen, ...
      |     'ninputs', ninputs, ...
      |     'max_accum', max_accum, ...
      |     'arith_type', arith_type, ...
      |     'in_bit_width', in_bit_width, ...
      |     'in_bin_pt', in_bin_pt, ...
      |     'out_bit_width', out_bit_width, ...
      |     'out_bin_pt', out_bin_pt, ...
      |     'register_din', register_din, ...
      |     'add_latency', add_latency, ...
      |     'bram_latency', bram_latency, ...
      |     'mux_latency', mux_latency);
      settings: AttributesFormatString=|
      | vector length=8, inputs=2
      | max accumulations=2^27
  wires:
  Constant/1 -> rst/1
  rst/1 -> cnt_rst/1, core_rst/1, sync_rst/1
  core_rst/1 -> posedge2/1
  cnt_rst/1 -> posedge1/1
  Constant2/1 -> dest_port/1
  Constant1/1 -> dest_ip/1
  dest_ip/1 -> gbe0/4
  dest_port/1 -> Slice/1
  Slice/1 -> gbe0/5
  gbe0/6 -> Terminator/1
  gbe0/7 -> Terminator1/1
  gbe0/8 -> Terminator2/1
  gbe0/9 -> Terminator3/1
  gbe0/10 -> Terminator4/1
  gbe0/11 -> Terminator5/1
  Constant3/1 -> gbe0/7
  gbe0/1 -> gbe0_linkup/1
  gbe0/2 -> gbe0_rx/1
  gbe0/3 -> gbe0_tx/1
  gbe0/4 -> negedge_delay/1
  negedge_delay/1 -> gbe0_full/1
  gbe0/5 -> negedge_delay1/1
  negedge_delay1/1 -> gbe0_overflow/1, led0_gbe0_overflow/1
  payload_cnt5/1 -> Slice6/1, gbe0_tx_cnt/1
  Slice6/1 -> led0_gbe0_tx/1
  [cnt_rst] -> payload_cnt5/1
  led0_gbe0_tx/1 -> Terminator16/1
  gbe0_tx_cnt/1 -> Terminator17/1
  gbe0_linkup/1 -> Terminator6/1
  gbe0_rx/1 -> Terminator7/1
  gbe0_tx/1 -> Terminator8/1
  gbe0_full/1 -> Terminator9/1
  gbe0_overflow/1 -> Terminator10/1
  led0_gbe0_overflow/1 -> Terminator11/1
  Constant4/1 -> gbe0/8
  gbe0/12 -> Terminator12/1
  sync_rst/1 -> posedge/1
  posedge/1 -> [sync_rst]
  posedge1/1 -> [cnt_rst]
  posedge2/1 -> [core_rst]
  Constant7/1 -> pkt_chan_sel/1
  pkt_chan_sel/1 -> pipeline1/1(d)
  Gateway Out4/1 -> Scope2/1
  Gateway Out5/1 -> Scope2/2
  Gateway Out6/1 -> Scope2/3
  [pkt_en] -> Mux/1
  Constant6/1 -> Mux/2
  Mux/1 -> gbe0/3
  [pkt_data] -> gbe0/2
  Constant8/1 -> pkt_payload_len/1
  pkt_payload_len/1 -> pipeline2/1(d)
  [bitcon_data] -> Delay2/1
  [bitcon_valid] -> Delay3/1
  [head_new_pkt] -> Gateway Out6/1
  [pkt_data_valid] -> Mux/3
  [pkt_eof] -> gbe0/6
  [head_new_pkt] -> payload_cnt5/2
  Gateway Out1/1 -> Scope1/1
  Gateway Out2/1 -> Scope1/2
  Gateway Out3/1 -> Scope1/3
  [pkt_data] -> Gateway Out1/1
  [pkt_data_valid] -> Gateway Out2/1
  [pkt_eof] -> Gateway Out3/1
  Delay2/1 -> Gateway Out4/1
  Delay3/1 -> Gateway Out5/1
  [pps] -> sync/2(pps_in)
  sync/1(Out1) -> [sync_gen], Gateway Out7/1
  [cnt_rst] -> sync/1(rst_in)
  Gateway Out7/1 -> Scope3/1
  Constant18/1 -> fft_shift/1
  fft_shift/1 -> pipeline28/1(d)
  pipeline28/1(q) -> [fft_shift]
  [sync_gen] -> fft_11x_0/1
  Band-Limited White Noise/1 -> adc0/1, adc0/2
  Pulse Generator/1 -> adc0/3
  Constant10/1 -> adc0/4
  adc0/9 -> or0/1
  adc0/10 -> or0/2
  adc0/11 -> or0/3
  adc0/12 -> or0/4
  adc0/13 -> or1/1
  adc0/14 -> or1/2
  adc0/15 -> or1/3
  adc0/16 -> or1/4
  adc0/17 -> Arnie9/1
  or0/1 -> [adc0_clip]
  or1/1 -> [pps]
  adc0/1 -> pl/1(In1)
  adc0/2 -> pl/2(In2)
  adc0/3 -> pl/3(In3)
  adc0/4 -> pl/4(In4)
  adc0/5 -> pl/5(In5)
  adc0/6 -> pl/6(In6)
  adc0/7 -> pl/7(In7)
  adc0/8 -> pl/8(In8)
  pl/1(Out1) -> [adc0_i0]
  pl/2(Out2) -> [adc0_i1]
  pl/3(Out3) -> [adc0_i2]
  pl/4(Out4) -> [adc0_i3]
  pl/5(Out5) -> [adc0_q0]
  pl/6(Out6) -> [adc0_q1]
  pl/7(Out7) -> [adc0_q2]
  pl/8(Out8) -> [adc0_q3]
  [mux_i0] -> Reinterpret8/1
  pipeline43/1(q) -> o_fft_of/1
  o_fft_of/1 -> Terminator13/1
  pipeline44/1(q) -> o_adc0_clip/1
  [adc0_clip] -> pulse_ext3/1
  o_adc0_clip/1 -> Terminator15/1
  pulse_ext3/1 -> pipeline44/1(d)
  [fft_of] -> pulse_ext/1
  pulse_ext/1 -> pipeline43/1(d)
  pps_cntr/1 -> pipeline46/1(d)
  pipeline46/1(q) -> o_pps_cnt/1
  o_pps_cnt/1 -> Terminator14/1
  [cnt_rst] -> pps_cntr/1
  posedge3/1 -> pps_cntr/2
  [pps] -> posedge3/1
  pipeline1/1(q) -> [chan_sel]
  pipeline2/1(q) -> [payload_len]
  Constant5/1 -> pkt_enable/1
  pkt_enable/1 -> pipeline3/1(d)
  pipeline3/1(q) -> Slice1/1
  Slice1/1 -> [pkt_en]
  [core_rst] -> Logical1/2
  [pkt_en] -> posedge4/1
  Logical1/1 -> gbe0/1
  posedge4/1 -> Logical1/1
  Constant14/1 -> pipeline47/1(d)
  pipeline47/1(q) -> gpio_a_oe/1
  gpio_a_oe/1 -> Terminator18/1
  pipeline48/1(q) -> gpio_a_led0/1
  gpio_a_led0/1 -> Terminator19/1
  [pps] -> pulse_ext2/1
  pulse_ext2/1 -> pipeline48/1(d)
  fft_11x_0/3 -> [fft0_out1]
  fft_11x_0/4 -> [fft_of]
  fft_11x_0/2 -> [fft0_out0]
  fft_11x_0/1 -> [fft0_sync_out]
  Convert/1 -> fft_11x_0/3
  Convert1/1 -> fft_11x_0/4
  Convert2/1 -> fft_11x_0/5
  Convert3/1 -> fft_11x_0/6
  [fft_shift] -> fft_11x_0/2
  [fft0_sync_out] -> Delay7/1
  [fft0_out0] -> cplx_quant/1(in)
  [fft0_out1] -> cplx_quant/2(in1)
  Constant16/1 -> acc_len_test/1
  acc_len_test/1 -> pipeline14/1(d)
  pipeline14/1(q) -> vacc1/3(acc_len)
  cram5/1(Out) -> vacc1/2(din)
  buf_reord1/2(sync_out) -> pipeline22/1(d)
  buf_reord1/3(valid) -> pipeline17/1(d)
  pipeline22/1(q) -> snap_x_s/2(trig)
  pipeline17/1(q) -> snap_x_s/3(we)
  posedge7/1 -> buf_reord1/1(trig)
  pipeline18/1(q) -> buf_reord1/2(even_in)
  pipeline21/1(q) -> buf_reord1/3(odd_in)
  buf_reord1/1(dout) -> pipeline15/1(d)
  pipeline15/1(q) -> snap_x_s/1(din)
  vacc1/2(dout) -> uncram1/1(In)
  vacc1/1(valid) -> posedge7/1
  uncram1/1(Out1) -> pipeline18/1(d)
  uncram1/2(Out2) -> pipeline21/1(d)
  Delay7/1 -> [quant_s_sync]
  cplx_quant/1(out) -> [quant_s_xx0]
  cplx_quant/2(out1) -> [quant_s_xx1]
  [quant_s_sync] -> Delay6/1
  [quant_s_xx0] -> power_dsp48e2/1
  [quant_s_xx1] -> power_dsp48e3/1
  power_dsp48e2/1 -> cram5/1(In1)
  power_dsp48e3/1 -> cram5/2(In2)
  Delay6/1 -> vacc1/1(sync)
  [cnt_rst] -> noise_source/1
  noise_source/1 -> Reinterpret/1
  noise_source/2 -> Reinterpret1/1
  noise_source/3 -> Reinterpret2/1
  noise_source/4 -> Reinterpret3/1
  noise_source/5 -> Reinterpret4/1
  noise_source/6 -> Reinterpret5/1
  noise_source/7 -> Reinterpret6/1
  noise_source/8 -> Reinterpret7/1
  Reinterpret/1 -> Arnie1/1
  Reinterpret1/1 -> Arnie2/1
  Reinterpret2/1 -> Arnie3/1
  Reinterpret3/1 -> Arnie4/1
  Reinterpret4/1 -> [m0]
  Reinterpret5/1 -> [m1]
  Reinterpret6/1 -> [m2]
  Reinterpret7/1 -> [m3]
  [mux_sel] -> adc_mux/1(mux_sel)
  [adc0_i0] -> adc_mux/2(i0)
  [adc0_i1] -> adc_mux/3(i1)
  [adc0_i2] -> adc_mux/4(i2)
  [adc0_i3] -> adc_mux/5(i3)
  adc_mux/1(Out0) -> [mux_i0]
  adc_mux/2(Out2) -> [mux_i1]
  adc_mux/3(Out3) -> [mux_i2]
  adc_mux/4(Out4) -> [mux_i3]
  [m0] -> adc_mux/6(n0)
  [m1] -> adc_mux/7(n1)
  [m2] -> adc_mux/8(n2)
  [m3] -> adc_mux/9(n3)
  [mux_i1] -> Reinterpret9/1
  [mux_i2] -> Reinterpret10/1
  [mux_i3] -> Reinterpret11/1
  mux_sel/1 -> pipeline50/1(d)
  Constant13/1 -> mux_sel/1
  Slice3/1 -> [mux_sel]
  pipeline50/1(q) -> Slice3/1, Slice5/1
  Slice5/1 -> [mux_sel2]
  Constant17/1 -> snap_mux/3(we), snap_mux/2(trig)
  [mux_i0] -> cram1/1
  [mux_i1] -> cram1/2
  [mux_i2] -> cram1/3
  [mux_i3] -> cram1/4
  cram1/1 -> snap_mux/1(din)
  bit_buffer/2(data_out) -> bit_concat/2(data_in)
  bit_concat/2(data_out) -> [bitcon_data]
  bit_concat/1(valid_out) -> [bitcon_valid]
  [chan_sel] -> chan_sel/2(chan_sel)
  chan_sel/1(sync_out) -> bit_buffer/1(sync)
  chan_sel/2(valid_out) -> bit_buffer/2(valid_in)
  chan_sel/3(d_out) -> bit_buffer/3(data_in)
  bit_buffer/1(valid_out) -> bit_concat/1(valid_in)
  [quant_s_xx0] -> chan_sel/3(d_in0)
  [quant_s_xx1] -> chan_sel/4(d_in1)
  [quant_s_sync] -> chan_sel/1(sync)
  [payload_len] -> header/2(payload_len)
  [bitcon_valid] -> header/3(data_valid)
  header/1(head_out) -> [head_out]
  header/2(new_pkt) -> [head_new_pkt]
  [pkt_en] -> posedge5/1
  posedge5/1 -> Logical2/1
  [cnt_rst] -> Logical2/2
  Logical2/1 -> header/1(rst)
  [head_out] -> packetizer/1(header_in)
  [bitcon_data] -> Delay/1
  [head_new_pkt] -> packetizer/3(new_pkt)
  packetizer/1(data_out) -> [pkt_data]
  packetizer/2(valid) -> [pkt_data_valid]
  packetizer/3(eof) -> [pkt_eof]
  Delay/1 -> packetizer/2(data_in)
  Delay1/1 -> packetizer/4(data_valid)
  [pkt_en] -> Logical/2
  [bitcon_valid] -> Logical/1
  Logical/1 -> Delay1/1
  Reinterpret8/1 -> Convert/1
  Reinterpret9/1 -> Convert1/1
  Reinterpret10/1 -> Convert2/1
  Reinterpret11/1 -> Convert3/1
